simulink library "Garbage"  (R2023a) (continued)
== system Garbage/Text_Display_1x16/Character_Table/Characters(Q..ARROW_UP) ==
  Address_3_In(1b) : Inport
  Address_2_In(1b) : Inport  Port=2
  Address_1_In(1b) : Inport  Port=3
  Address_0_In(1b) : Inport  Port=4
  Byte_Multiplexer_16_to_1(Byte_0) : Byte_Multiplexers_Lib/Byte_Multiplexers_Lib/Byte_Multiplexer_16_to_1
  Byte_Multiplexer_16_to_1(Byte_1) : Byte_Multiplexers_Lib/Byte_Multiplexers_Lib/Byte_Multiplexer_16_to_1
  Byte_Multiplexer_16_to_1(Byte_2) : Byte_Multiplexers_Lib/Byte_Multiplexers_Lib/Byte_Multiplexer_16_to_1
  Byte_Multiplexer_16_to_1(Byte_3) : Byte_Multiplexers_Lib/Byte_Multiplexers_Lib/Byte_Multiplexer_16_to_1
  Byte_Multiplexer_16_to_1(Byte_4) : Byte_Multiplexers_Lib/Byte_Multiplexers_Lib/Byte_Multiplexer_16_to_1
  Byte_Multiplexer_16_to_1(Byte_5) : Byte_Multiplexers_Lib/Byte_Multiplexers_Lib/Byte_Multiplexer_16_to_1
  Byte_Multiplexer_16_to_1(Byte_6) : Byte_Multiplexers_Lib/Byte_Multiplexers_Lib/Byte_Multiplexer_16_to_1
  Byte_Multiplexer_16_to_1(Byte_7) : Byte_Multiplexers_Lib/Byte_Multiplexers_Lib/Byte_Multiplexer_16_to_1
  Char_ARROW_UP : Subsystem
  Char_ASTERISK : Subsystem
  Char_BACKSLASH : Subsystem
  Char_COLON : Subsystem
  Char_CROSS : Subsystem
  Char_EQUAL : Subsystem
  Char_GREATER_THAN : Subsystem
  Char_LESS_THAN : Subsystem
  Char_MINUS : Subsystem
  Char_PLUS : Subsystem
  Char_Q : Subsystem
  Char_SEMICOLON : Subsystem
  Char_SLASH : Subsystem
  Char_X : Subsystem
  Char_Y : Subsystem
  Char_Z : Subsystem
  Row_7(1B) : Outport
  Row_6(1B) : Outport  Port=2
  Row_5(1B) : Outport  Port=3
  Row_4(1B) : Outport  Port=4
  Row_3(1B) : Outport  Port=5
  Row_2(1B) : Outport  Port=6
  Row_1(1B) : Outport  Port=7
  Row_0(1B) : Outport  Port=8
  wires:
  Address_3_In(1b)/1 -> Byte_Multiplexer_16_to_1(Byte_0)/1, Byte_Multiplexer_16_to_1(Byte_1)/1, Byte_Multiplexer_16_to_1(Byte_2)/1, Byte_Multiplexer_16_to_1(Byte_3)/1, Byte_Multiplexer_16_to_1(Byte_4)/1, Byte_Multiplexer_16_to_1(Byte_5)/1, Byte_Multiplexer_16_to_1(Byte_6)/1, Byte_Multiplexer_16_to_1(Byte_7)/1
  Address_2_In(1b)/1 -> Byte_Multiplexer_16_to_1(Byte_0)/2, Byte_Multiplexer_16_to_1(Byte_1)/2, Byte_Multiplexer_16_to_1(Byte_2)/2, Byte_Multiplexer_16_to_1(Byte_3)/2, Byte_Multiplexer_16_to_1(Byte_4)/2, Byte_Multiplexer_16_to_1(Byte_5)/2, Byte_Multiplexer_16_to_1(Byte_6)/2, Byte_Multiplexer_16_to_1(Byte_7)/2
  Address_1_In(1b)/1 -> Byte_Multiplexer_16_to_1(Byte_0)/3, Byte_Multiplexer_16_to_1(Byte_1)/3, Byte_Multiplexer_16_to_1(Byte_2)/3, Byte_Multiplexer_16_to_1(Byte_3)/3, Byte_Multiplexer_16_to_1(Byte_4)/3, Byte_Multiplexer_16_to_1(Byte_5)/3, Byte_Multiplexer_16_to_1(Byte_6)/3, Byte_Multiplexer_16_to_1(Byte_7)/3
  Address_0_In(1b)/1 -> Byte_Multiplexer_16_to_1(Byte_0)/4, Byte_Multiplexer_16_to_1(Byte_1)/4, Byte_Multiplexer_16_to_1(Byte_2)/4, Byte_Multiplexer_16_to_1(Byte_3)/4, Byte_Multiplexer_16_to_1(Byte_4)/4, Byte_Multiplexer_16_to_1(Byte_5)/4, Byte_Multiplexer_16_to_1(Byte_6)/4, Byte_Multiplexer_16_to_1(Byte_7)/4
  Byte_Multiplexer_16_to_1(Byte_0)/1 -> Row_0(1B)/1
  Byte_Multiplexer_16_to_1(Byte_1)/1 -> Row_1(1B)/1
  Byte_Multiplexer_16_to_1(Byte_2)/1 -> Row_2(1B)/1
  Byte_Multiplexer_16_to_1(Byte_3)/1 -> Row_3(1B)/1
  Byte_Multiplexer_16_to_1(Byte_4)/1 -> Row_4(1B)/1
  Byte_Multiplexer_16_to_1(Byte_5)/1 -> Row_5(1B)/1
  Byte_Multiplexer_16_to_1(Byte_6)/1 -> Row_6(1B)/1
  Byte_Multiplexer_16_to_1(Byte_7)/1 -> Row_7(1B)/1
  Char_ARROW_UP/8(Row_0(1B)) -> Byte_Multiplexer_16_to_1(Byte_0)/5
  Char_GREATER_THAN/8(Row_0(1B)) -> Byte_Multiplexer_16_to_1(Byte_0)/6
  Char_LESS_THAN/8(Row_0(1B)) -> Byte_Multiplexer_16_to_1(Byte_0)/7
  Char_EQUAL/8(Row_0(1B)) -> Byte_Multiplexer_16_to_1(Byte_0)/8
  Char_BACKSLASH/8(Row_0(1B)) -> Byte_Multiplexer_16_to_1(Byte_0)/9
  Char_SLASH/8(Row_0(1B)) -> Byte_Multiplexer_16_to_1(Byte_0)/10
  Char_SEMICOLON/8(Row_0(1B)) -> Byte_Multiplexer_16_to_1(Byte_0)/11
  Char_COLON/8(Row_0(1B)) -> Byte_Multiplexer_16_to_1(Byte_0)/12
  Char_ASTERISK/8(Row_0(1B)) -> Byte_Multiplexer_16_to_1(Byte_0)/13
  Char_CROSS/8(Row_0(1B)) -> Byte_Multiplexer_16_to_1(Byte_0)/14
  Char_MINUS/8(Row_0(1B)) -> Byte_Multiplexer_16_to_1(Byte_0)/15
  Char_PLUS/8(Row_0(1B)) -> Byte_Multiplexer_16_to_1(Byte_0)/16
  Char_Z/8(Row_0(1B)) -> Byte_Multiplexer_16_to_1(Byte_0)/17
  Char_Y/8(Row_0(1B)) -> Byte_Multiplexer_16_to_1(Byte_0)/18
  Char_X/8(Row_0(1B)) -> Byte_Multiplexer_16_to_1(Byte_0)/19
  Char_Q/8(Row_0(1B)) -> Byte_Multiplexer_16_to_1(Byte_0)/20
  Char_ARROW_UP/7(Row_1(1B)) -> Byte_Multiplexer_16_to_1(Byte_1)/5
  Char_GREATER_THAN/7(Row_1(1B)) -> Byte_Multiplexer_16_to_1(Byte_1)/6
  Char_LESS_THAN/7(Row_1(1B)) -> Byte_Multiplexer_16_to_1(Byte_1)/7
  Char_BACKSLASH/7(Row_1(1B)) -> Byte_Multiplexer_16_to_1(Byte_1)/9
  Char_SLASH/7(Row_1(1B)) -> Byte_Multiplexer_16_to_1(Byte_1)/10
  Char_SEMICOLON/7(Row_1(1B)) -> Byte_Multiplexer_16_to_1(Byte_1)/11
  Char_COLON/7(Row_1(1B)) -> Byte_Multiplexer_16_to_1(Byte_1)/12
  Char_ASTERISK/7(Row_1(1B)) -> Byte_Multiplexer_16_to_1(Byte_1)/13
  Char_CROSS/7(Row_1(1B)) -> Byte_Multiplexer_16_to_1(Byte_1)/14
  Char_MINUS/7(Row_1(1B)) -> Byte_Multiplexer_16_to_1(Byte_1)/15
  Char_PLUS/7(Row_1(1B)) -> Byte_Multiplexer_16_to_1(Byte_1)/16
  Char_Z/7(Row_1(1B)) -> Byte_Multiplexer_16_to_1(Byte_1)/17
  Char_Y/7(Row_1(1B)) -> Byte_Multiplexer_16_to_1(Byte_1)/18
  Char_X/7(Row_1(1B)) -> Byte_Multiplexer_16_to_1(Byte_1)/19
  Char_Q/7(Row_1(1B)) -> Byte_Multiplexer_16_to_1(Byte_1)/20
  Char_ARROW_UP/6(Row_2(1B)) -> Byte_Multiplexer_16_to_1(Byte_2)/5
  Char_GREATER_THAN/6(Row_2(1B)) -> Byte_Multiplexer_16_to_1(Byte_2)/6
  Char_LESS_THAN/6(Row_2(1B)) -> Byte_Multiplexer_16_to_1(Byte_2)/7
  Char_EQUAL/6(Row_2(1B)) -> Byte_Multiplexer_16_to_1(Byte_2)/8
  Char_EQUAL/7(Row_1(1B)) -> Byte_Multiplexer_16_to_1(Byte_1)/8
  Char_BACKSLASH/6(Row_2(1B)) -> Byte_Multiplexer_16_to_1(Byte_2)/9
  Char_SLASH/6(Row_2(1B)) -> Byte_Multiplexer_16_to_1(Byte_2)/10
  Char_SEMICOLON/6(Row_2(1B)) -> Byte_Multiplexer_16_to_1(Byte_2)/11
  Char_COLON/6(Row_2(1B)) -> Byte_Multiplexer_16_to_1(Byte_2)/12
  Char_ASTERISK/6(Row_2(1B)) -> Byte_Multiplexer_16_to_1(Byte_2)/13
  Char_CROSS/6(Row_2(1B)) -> Byte_Multiplexer_16_to_1(Byte_2)/14
  Char_MINUS/6(Row_2(1B)) -> Byte_Multiplexer_16_to_1(Byte_2)/15
  Char_PLUS/6(Row_2(1B)) -> Byte_Multiplexer_16_to_1(Byte_2)/16
  Char_Z/6(Row_2(1B)) -> Byte_Multiplexer_16_to_1(Byte_2)/17
  Char_Y/6(Row_2(1B)) -> Byte_Multiplexer_16_to_1(Byte_2)/18
  Char_X/6(Row_2(1B)) -> Byte_Multiplexer_16_to_1(Byte_2)/19
  Char_Q/6(Row_2(1B)) -> Byte_Multiplexer_16_to_1(Byte_2)/20
  Char_ARROW_UP/5(Row_3(1B)) -> Byte_Multiplexer_16_to_1(Byte_3)/5
  Char_GREATER_THAN/5(Row_3(1B)) -> Byte_Multiplexer_16_to_1(Byte_3)/6
  Char_LESS_THAN/5(Row_3(1B)) -> Byte_Multiplexer_16_to_1(Byte_3)/7
  Char_EQUAL/5(Row_3(1B)) -> Byte_Multiplexer_16_to_1(Byte_3)/8
  Char_BACKSLASH/5(Row_3(1B)) -> Byte_Multiplexer_16_to_1(Byte_3)/9
  Char_SLASH/5(Row_3(1B)) -> Byte_Multiplexer_16_to_1(Byte_3)/10
  Char_SEMICOLON/5(Row_3(1B)) -> Byte_Multiplexer_16_to_1(Byte_3)/11
  Char_COLON/5(Row_3(1B)) -> Byte_Multiplexer_16_to_1(Byte_3)/12
  Char_CROSS/5(Row_3(1B)) -> Byte_Multiplexer_16_to_1(Byte_3)/14
  Char_ASTERISK/5(Row_3(1B)) -> Byte_Multiplexer_16_to_1(Byte_3)/13
  Char_MINUS/5(Row_3(1B)) -> Byte_Multiplexer_16_to_1(Byte_3)/15
  Char_PLUS/5(Row_3(1B)) -> Byte_Multiplexer_16_to_1(Byte_3)/16
  Char_Z/5(Row_3(1B)) -> Byte_Multiplexer_16_to_1(Byte_3)/17
  Char_Y/5(Row_3(1B)) -> Byte_Multiplexer_16_to_1(Byte_3)/18
  Char_X/5(Row_3(1B)) -> Byte_Multiplexer_16_to_1(Byte_3)/19
  Char_Q/5(Row_3(1B)) -> Byte_Multiplexer_16_to_1(Byte_3)/20
  Char_ARROW_UP/4(Row_4(1B)) -> Byte_Multiplexer_16_to_1(Byte_4)/5
  Char_GREATER_THAN/4(Row_4(1B)) -> Byte_Multiplexer_16_to_1(Byte_4)/6
  Char_LESS_THAN/4(Row_4(1B)) -> Byte_Multiplexer_16_to_1(Byte_4)/7
  Char_EQUAL/4(Row_4(1B)) -> Byte_Multiplexer_16_to_1(Byte_4)/8
  Char_BACKSLASH/4(Row_4(1B)) -> Byte_Multiplexer_16_to_1(Byte_4)/9
  Char_SLASH/4(Row_4(1B)) -> Byte_Multiplexer_16_to_1(Byte_4)/10
  Char_SEMICOLON/4(Row_4(1B)) -> Byte_Multiplexer_16_to_1(Byte_4)/11
  Char_COLON/4(Row_4(1B)) -> Byte_Multiplexer_16_to_1(Byte_4)/12
  Char_ASTERISK/4(Row_4(1B)) -> Byte_Multiplexer_16_to_1(Byte_4)/13
  Char_CROSS/4(Row_4(1B)) -> Byte_Multiplexer_16_to_1(Byte_4)/14
  Char_MINUS/4(Row_4(1B)) -> Byte_Multiplexer_16_to_1(Byte_4)/15
  Char_PLUS/4(Row_4(1B)) -> Byte_Multiplexer_16_to_1(Byte_4)/16
  Char_Z/4(Row_4(1B)) -> Byte_Multiplexer_16_to_1(Byte_4)/17
  Char_Y/4(Row_4(1B)) -> Byte_Multiplexer_16_to_1(Byte_4)/18
  Char_X/4(Row_4(1B)) -> Byte_Multiplexer_16_to_1(Byte_4)/19
  Char_Q/4(Row_4(1B)) -> Byte_Multiplexer_16_to_1(Byte_4)/20
  Char_ARROW_UP/3(Row_5(1B)) -> Byte_Multiplexer_16_to_1(Byte_5)/5
  Char_GREATER_THAN/3(Row_5(1B)) -> Byte_Multiplexer_16_to_1(Byte_5)/6
  Char_LESS_THAN/3(Row_5(1B)) -> Byte_Multiplexer_16_to_1(Byte_5)/7
  Char_EQUAL/3(Row_5(1B)) -> Byte_Multiplexer_16_to_1(Byte_5)/8
  Char_BACKSLASH/3(Row_5(1B)) -> Byte_Multiplexer_16_to_1(Byte_5)/9
  Char_SLASH/3(Row_5(1B)) -> Byte_Multiplexer_16_to_1(Byte_5)/10
  Char_SEMICOLON/3(Row_5(1B)) -> Byte_Multiplexer_16_to_1(Byte_5)/11
  Char_COLON/3(Row_5(1B)) -> Byte_Multiplexer_16_to_1(Byte_5)/12
  Char_ASTERISK/3(Row_5(1B)) -> Byte_Multiplexer_16_to_1(Byte_5)/13
  Char_CROSS/3(Row_5(1B)) -> Byte_Multiplexer_16_to_1(Byte_5)/14
  Char_MINUS/3(Row_5(1B)) -> Byte_Multiplexer_16_to_1(Byte_5)/15
  Char_PLUS/3(Row_5(1B)) -> Byte_Multiplexer_16_to_1(Byte_5)/16
  Char_Z/3(Row_5(1B)) -> Byte_Multiplexer_16_to_1(Byte_5)/17
  Char_Y/3(Row_5(1B)) -> Byte_Multiplexer_16_to_1(Byte_5)/18
  Char_X/3(Row_5(1B)) -> Byte_Multiplexer_16_to_1(Byte_5)/19
  Char_Q/3(Row_5(1B)) -> Byte_Multiplexer_16_to_1(Byte_5)/20
  Char_GREATER_THAN/2(Row_6(1B)) -> Byte_Multiplexer_16_to_1(Byte_6)/6
  Char_LESS_THAN/2(Row_6(1B)) -> Byte_Multiplexer_16_to_1(Byte_6)/7
  Char_EQUAL/2(Row_6(1B)) -> Byte_Multiplexer_16_to_1(Byte_6)/8
  Char_BACKSLASH/2(Row_6(1B)) -> Byte_Multiplexer_16_to_1(Byte_6)/9
  Char_SLASH/2(Row_6(1B)) -> Byte_Multiplexer_16_to_1(Byte_6)/10
  Char_SEMICOLON/2(Row_6(1B)) -> Byte_Multiplexer_16_to_1(Byte_6)/11
  Char_COLON/2(Row_6(1B)) -> Byte_Multiplexer_16_to_1(Byte_6)/12
  Char_ASTERISK/2(Row_6(1B)) -> Byte_Multiplexer_16_to_1(Byte_6)/13
  Char_CROSS/2(Row_6(1B)) -> Byte_Multiplexer_16_to_1(Byte_6)/14
  Char_MINUS/2(Row_6(1B)) -> Byte_Multiplexer_16_to_1(Byte_6)/15
  Char_PLUS/2(Row_6(1B)) -> Byte_Multiplexer_16_to_1(Byte_6)/16
  Char_Z/2(Row_6(1B)) -> Byte_Multiplexer_16_to_1(Byte_6)/17
  Char_Y/2(Row_6(1B)) -> Byte_Multiplexer_16_to_1(Byte_6)/18
  Char_X/2(Row_6(1B)) -> Byte_Multiplexer_16_to_1(Byte_6)/19
  Char_Q/2(Row_6(1B)) -> Byte_Multiplexer_16_to_1(Byte_6)/20
  Char_ARROW_UP/2(Row_6(1B)) -> Byte_Multiplexer_16_to_1(Byte_6)/5
  Char_Q/1(Row_7(1B)) -> Byte_Multiplexer_16_to_1(Byte_7)/20
  Char_X/1(Row_7(1B)) -> Byte_Multiplexer_16_to_1(Byte_7)/19
  Char_Y/1(Row_7(1B)) -> Byte_Multiplexer_16_to_1(Byte_7)/18
  Char_Z/1(Row_7(1B)) -> Byte_Multiplexer_16_to_1(Byte_7)/17
  Char_PLUS/1(Row_7(1B)) -> Byte_Multiplexer_16_to_1(Byte_7)/16
  Char_MINUS/1(Row_7(1B)) -> Byte_Multiplexer_16_to_1(Byte_7)/15
  Char_CROSS/1(Row_7(1B)) -> Byte_Multiplexer_16_to_1(Byte_7)/14
  Char_ASTERISK/1(Row_7(1B)) -> Byte_Multiplexer_16_to_1(Byte_7)/13
  Char_COLON/1(Row_7(1B)) -> Byte_Multiplexer_16_to_1(Byte_7)/12
  Char_SEMICOLON/1(Row_7(1B)) -> Byte_Multiplexer_16_to_1(Byte_7)/11
  Char_SLASH/1(Row_7(1B)) -> Byte_Multiplexer_16_to_1(Byte_7)/10
  Char_BACKSLASH/1(Row_7(1B)) -> Byte_Multiplexer_16_to_1(Byte_7)/9
  Char_EQUAL/1(Row_7(1B)) -> Byte_Multiplexer_16_to_1(Byte_7)/8
  Char_LESS_THAN/1(Row_7(1B)) -> Byte_Multiplexer_16_to_1(Byte_7)/7
  Char_GREATER_THAN/1(Row_7(1B)) -> Byte_Multiplexer_16_to_1(Byte_7)/6
  Char_ARROW_UP/1(Row_7(1B)) -> Byte_Multiplexer_16_to_1(Byte_7)/5

== system Garbage/Text_Display_1x16/Character_Table/Characters(0..F)/Char_0 ==
  Byte_01 : Constant  Value=c.char_0(:, 2)  OutDataTypeStr=uint8
  Byte_02 : Constant  Value=c.char_0(:, 3)  OutDataTypeStr=uint8
  Byte_03 : Constant  Value=c.char_0(:, 4)  OutDataTypeStr=uint8
  Byte_04 : Constant  Value=c.char_0(:, 5)  OutDataTypeStr=uint8
  Byte_05 : Constant  Value=c.char_0(:, 6)  OutDataTypeStr=uint8
  Byte_06 : Constant  Value=c.char_0(:, 7)  OutDataTypeStr=uint8
  Byte_07 : Constant  Value=c.char_0(:, 8)  OutDataTypeStr=uint8
  Byte_1 : Constant  Value=c.char_0(:, 1)  OutDataTypeStr=uint8
  Row_7(1B) : Outport
  Row_6(1B) : Outport  Port=2
  Row_5(1B) : Outport  Port=3
  Row_4(1B) : Outport  Port=4
  Row_3(1B) : Outport  Port=5
  Row_2(1B) : Outport  Port=6
  Row_1(1B) : Outport  Port=7
  Row_0(1B) : Outport  Port=8
  wires:
  Byte_02/1 -> Row_5(1B)/1
  Byte_1/1 -> Row_7(1B)/1
  Byte_06/1 -> Row_1(1B)/1
  Byte_03/1 -> Row_4(1B)/1
  Byte_07/1 -> Row_0(1B)/1
  Byte_01/1 -> Row_6(1B)/1
  Byte_04/1 -> Row_3(1B)/1
  Byte_05/1 -> Row_2(1B)/1

== system Garbage/Text_Display_1x16/Character_Table/Characters(0..F)/Char_1 ==
  Byte_01 : Constant  Value=c.char(2, 1)  OutDataTypeStr=uint8
  Byte_02 : Constant  Value=c.char(3, 1)  OutDataTypeStr=uint8
  Byte_03 : Constant  Value=c.char(4, 1)  OutDataTypeStr=uint8
  Byte_04 : Constant  Value=c.char(5, 1)  OutDataTypeStr=uint8
  Byte_05 : Constant  Value=c.char(6, 1)  OutDataTypeStr=uint8
  Byte_06 : Constant  Value=c.char(7, 1)  OutDataTypeStr=uint8
  Byte_07 : Constant  Value=c.char(8, 1)  OutDataTypeStr=uint8
  Byte_1 : Constant  Value=c.char(1, 1)  OutDataTypeStr=uint8
  Row_7(1B) : Outport
  Row_6(1B) : Outport  Port=2
  Row_5(1B) : Outport  Port=3
  Row_4(1B) : Outport  Port=4
  Row_3(1B) : Outport  Port=5
  Row_2(1B) : Outport  Port=6
  Row_1(1B) : Outport  Port=7
  Row_0(1B) : Outport  Port=8
  wires:
  Byte_02/1 -> Row_5(1B)/1
  Byte_1/1 -> Row_7(1B)/1
  Byte_06/1 -> Row_1(1B)/1
  Byte_03/1 -> Row_4(1B)/1
  Byte_07/1 -> Row_0(1B)/1
  Byte_01/1 -> Row_6(1B)/1
  Byte_04/1 -> Row_3(1B)/1
  Byte_05/1 -> Row_2(1B)/1

== system Garbage/Text_Display_1x16/Character_Table/Characters(0..F)/Char_2 ==
  Byte_01 : Constant  Value=c.char(2, 2)  OutDataTypeStr=uint8
  Byte_02 : Constant  Value=c.char(3, 2)  OutDataTypeStr=uint8
  Byte_03 : Constant  Value=c.char(4, 2)  OutDataTypeStr=uint8
  Byte_04 : Constant  Value=c.char(5, 2)  OutDataTypeStr=uint8
  Byte_05 : Constant  Value=c.char(6, 2)  OutDataTypeStr=uint8
  Byte_06 : Constant  Value=c.char(7, 2)  OutDataTypeStr=uint8
  Byte_07 : Constant  Value=c.char(8, 2)  OutDataTypeStr=uint8
  Byte_1 : Constant  Value=c.char(1, 2)  OutDataTypeStr=uint8
  Row_7(1B) : Outport
  Row_6(1B) : Outport  Port=2
  Row_5(1B) : Outport  Port=3
  Row_4(1B) : Outport  Port=4
  Row_3(1B) : Outport  Port=5
  Row_2(1B) : Outport  Port=6
  Row_1(1B) : Outport  Port=7
  Row_0(1B) : Outport  Port=8
  wires:
  Byte_02/1 -> Row_5(1B)/1
  Byte_1/1 -> Row_7(1B)/1
  Byte_06/1 -> Row_1(1B)/1
  Byte_03/1 -> Row_4(1B)/1
  Byte_07/1 -> Row_0(1B)/1
  Byte_01/1 -> Row_6(1B)/1
  Byte_04/1 -> Row_3(1B)/1
  Byte_05/1 -> Row_2(1B)/1

== system Garbage/Text_Display_1x16/Character_Table/Characters(0..F)/Char_3 ==
  Byte_01 : Constant  Value=c.char(2, 3)  OutDataTypeStr=uint8
  Byte_02 : Constant  Value=c.char(3, 3)  OutDataTypeStr=uint8
  Byte_03 : Constant  Value=c.char(4, 3)  OutDataTypeStr=uint8
  Byte_04 : Constant  Value=c.char(5, 3)  OutDataTypeStr=uint8
  Byte_05 : Constant  Value=c.char(6, 3)  OutDataTypeStr=uint8
  Byte_06 : Constant  Value=c.char(7, 3)  OutDataTypeStr=uint8
  Byte_07 : Constant  Value=c.char(8, 3)  OutDataTypeStr=uint8
  Byte_1 : Constant  Value=c.char(1, 3)  OutDataTypeStr=uint8
  Row_7(1B) : Outport
  Row_6(1B) : Outport  Port=2
  Row_5(1B) : Outport  Port=3
  Row_4(1B) : Outport  Port=4
  Row_3(1B) : Outport  Port=5
  Row_2(1B) : Outport  Port=6
  Row_1(1B) : Outport  Port=7
  Row_0(1B) : Outport  Port=8
  wires:
  Byte_02/1 -> Row_5(1B)/1
  Byte_1/1 -> Row_7(1B)/1
  Byte_06/1 -> Row_1(1B)/1
  Byte_03/1 -> Row_4(1B)/1
  Byte_07/1 -> Row_0(1B)/1
  Byte_01/1 -> Row_6(1B)/1
  Byte_04/1 -> Row_3(1B)/1
  Byte_05/1 -> Row_2(1B)/1

== system Garbage/Text_Display_1x16/Character_Table/Characters(0..F)/Char_4 ==
  Byte_01 : Constant  Value=c.char(2, 4)  OutDataTypeStr=uint8
  Byte_02 : Constant  Value=c.char(3, 4)  OutDataTypeStr=uint8
  Byte_03 : Constant  Value=c.char(4, 4)  OutDataTypeStr=uint8
  Byte_04 : Constant  Value=c.char(5, 4)  OutDataTypeStr=uint8
  Byte_05 : Constant  Value=c.char(6, 4)  OutDataTypeStr=uint8
  Byte_06 : Constant  Value=c.char(7, 4)  OutDataTypeStr=uint8
  Byte_07 : Constant  Value=c.char(8, 4)  OutDataTypeStr=uint8
  Byte_1 : Constant  Value=c.char(1, 4)  OutDataTypeStr=uint8
  Row_7(1B) : Outport
  Row_6(1B) : Outport  Port=2
  Row_5(1B) : Outport  Port=3
  Row_4(1B) : Outport  Port=4
  Row_3(1B) : Outport  Port=5
  Row_2(1B) : Outport  Port=6
  Row_1(1B) : Outport  Port=7
  Row_0(1B) : Outport  Port=8
  wires:
  Byte_02/1 -> Row_5(1B)/1
  Byte_1/1 -> Row_7(1B)/1
  Byte_06/1 -> Row_1(1B)/1
  Byte_03/1 -> Row_4(1B)/1
  Byte_07/1 -> Row_0(1B)/1
  Byte_01/1 -> Row_6(1B)/1
  Byte_04/1 -> Row_3(1B)/1
  Byte_05/1 -> Row_2(1B)/1

== system Garbage/Text_Display_1x16/Character_Table/Characters(0..F)/Char_5 ==
  Byte_01 : Constant  Value=c.char(2, 5)  OutDataTypeStr=uint8
  Byte_02 : Constant  Value=c.char(3, 5)  OutDataTypeStr=uint8
  Byte_03 : Constant  Value=c.char(4, 5)  OutDataTypeStr=uint8
  Byte_04 : Constant  Value=c.char(5, 5)  OutDataTypeStr=uint8
  Byte_05 : Constant  Value=c.char(6, 5)  OutDataTypeStr=uint8
  Byte_06 : Constant  Value=c.char(7, 5)  OutDataTypeStr=uint8
  Byte_07 : Constant  Value=c.char(8, 5)  OutDataTypeStr=uint8
  Byte_1 : Constant  Value=c.char(1, 5)  OutDataTypeStr=uint8
  Row_7(1B) : Outport
  Row_6(1B) : Outport  Port=2
  Row_5(1B) : Outport  Port=3
  Row_4(1B) : Outport  Port=4
  Row_3(1B) : Outport  Port=5
  Row_2(1B) : Outport  Port=6
  Row_1(1B) : Outport  Port=7
  Row_0(1B) : Outport  Port=8
  wires:
  Byte_02/1 -> Row_5(1B)/1
  Byte_1/1 -> Row_7(1B)/1
  Byte_06/1 -> Row_1(1B)/1
  Byte_03/1 -> Row_4(1B)/1
  Byte_07/1 -> Row_0(1B)/1
  Byte_01/1 -> Row_6(1B)/1
  Byte_04/1 -> Row_3(1B)/1
  Byte_05/1 -> Row_2(1B)/1

== system Garbage/Text_Display_1x16/Character_Table/Characters(0..F)/Char_6 ==
  Byte_01 : Constant  Value=c.char(2, 6)  OutDataTypeStr=uint8
  Byte_02 : Constant  Value=c.char(3, 6)  OutDataTypeStr=uint8
  Byte_03 : Constant  Value=c.char(4, 6)  OutDataTypeStr=uint8
  Byte_04 : Constant  Value=c.char(5, 6)  OutDataTypeStr=uint8
  Byte_05 : Constant  Value=c.char(6, 6)  OutDataTypeStr=uint8
  Byte_06 : Constant  Value=c.char(7, 6)  OutDataTypeStr=uint8
  Byte_07 : Constant  Value=c.char(8, 6)  OutDataTypeStr=uint8
  Byte_1 : Constant  Value=c.char(1, 6)  OutDataTypeStr=uint8
  Row_7(1B) : Outport
  Row_6(1B) : Outport  Port=2
  Row_5(1B) : Outport  Port=3
  Row_4(1B) : Outport  Port=4
  Row_3(1B) : Outport  Port=5
  Row_2(1B) : Outport  Port=6
  Row_1(1B) : Outport  Port=7
  Row_0(1B) : Outport  Port=8
  wires:
  Byte_02/1 -> Row_5(1B)/1
  Byte_1/1 -> Row_7(1B)/1
  Byte_06/1 -> Row_1(1B)/1
  Byte_03/1 -> Row_4(1B)/1
  Byte_07/1 -> Row_0(1B)/1
  Byte_01/1 -> Row_6(1B)/1
  Byte_04/1 -> Row_3(1B)/1
  Byte_05/1 -> Row_2(1B)/1

== system Garbage/Text_Display_1x16/Character_Table/Characters(0..F)/Char_7 ==
  Byte_01 : Constant  Value=c.char(2, 7)  OutDataTypeStr=uint8
  Byte_02 : Constant  Value=c.char(3, 7)  OutDataTypeStr=uint8
  Byte_03 : Constant  Value=c.char(4, 7)  OutDataTypeStr=uint8
  Byte_04 : Constant  Value=c.char(5, 7)  OutDataTypeStr=uint8
  Byte_05 : Constant  Value=c.char(6, 7)  OutDataTypeStr=uint8
  Byte_06 : Constant  Value=c.char(7, 7)  OutDataTypeStr=uint8
  Byte_07 : Constant  Value=c.char(8, 7)  OutDataTypeStr=uint8
  Byte_1 : Constant  Value=c.char(1, 7)  OutDataTypeStr=uint8
  Row_7(1B) : Outport
  Row_6(1B) : Outport  Port=2
  Row_5(1B) : Outport  Port=3
  Row_4(1B) : Outport  Port=4
  Row_3(1B) : Outport  Port=5
  Row_2(1B) : Outport  Port=6
  Row_1(1B) : Outport  Port=7
  Row_0(1B) : Outport  Port=8
  wires:
  Byte_02/1 -> Row_5(1B)/1
  Byte_1/1 -> Row_7(1B)/1
  Byte_06/1 -> Row_1(1B)/1
  Byte_03/1 -> Row_4(1B)/1
  Byte_07/1 -> Row_0(1B)/1
  Byte_01/1 -> Row_6(1B)/1
  Byte_04/1 -> Row_3(1B)/1
  Byte_05/1 -> Row_2(1B)/1

== system Garbage/Text_Display_1x16/Character_Table/Characters(0..F)/Char_8 ==
  Byte_01 : Constant  Value=c.char(2, 8)  OutDataTypeStr=uint8
  Byte_02 : Constant  Value=c.char(3, 8)  OutDataTypeStr=uint8
  Byte_03 : Constant  Value=c.char(4, 8)  OutDataTypeStr=uint8
  Byte_04 : Constant  Value=c.char(5, 8)  OutDataTypeStr=uint8
  Byte_05 : Constant  Value=c.char(6, 8)  OutDataTypeStr=uint8
  Byte_06 : Constant  Value=c.char(7, 8)  OutDataTypeStr=uint8
  Byte_07 : Constant  Value=c.char(8, 8)  OutDataTypeStr=uint8
  Byte_1 : Constant  Value=c.char(1, 8)  OutDataTypeStr=uint8
  Row_7(1B) : Outport
  Row_6(1B) : Outport  Port=2
  Row_5(1B) : Outport  Port=3
  Row_4(1B) : Outport  Port=4
  Row_3(1B) : Outport  Port=5
  Row_2(1B) : Outport  Port=6
  Row_1(1B) : Outport  Port=7
  Row_0(1B) : Outport  Port=8
  wires:
  Byte_02/1 -> Row_5(1B)/1
  Byte_1/1 -> Row_7(1B)/1
  Byte_06/1 -> Row_1(1B)/1
  Byte_03/1 -> Row_4(1B)/1
  Byte_07/1 -> Row_0(1B)/1
  Byte_01/1 -> Row_6(1B)/1
  Byte_04/1 -> Row_3(1B)/1
  Byte_05/1 -> Row_2(1B)/1

== system Garbage/Text_Display_1x16/Character_Table/Characters(0..F)/Char_9 ==
  Byte_01 : Constant  Value=c.char(2, 9)  OutDataTypeStr=uint8
  Byte_02 : Constant  Value=c.char(3, 9)  OutDataTypeStr=uint8
  Byte_03 : Constant  Value=c.char(4, 9)  OutDataTypeStr=uint8
  Byte_04 : Constant  Value=c.char(5, 9)  OutDataTypeStr=uint8
  Byte_05 : Constant  Value=c.char(6, 9)  OutDataTypeStr=uint8
  Byte_06 : Constant  Value=c.char(7, 9)  OutDataTypeStr=uint8
  Byte_07 : Constant  Value=c.char(8, 9)  OutDataTypeStr=uint8
  Byte_1 : Constant  Value=c.char(1, 9)  OutDataTypeStr=uint8
  Row_7(1B) : Outport
  Row_6(1B) : Outport  Port=2
  Row_5(1B) : Outport  Port=3
  Row_4(1B) : Outport  Port=4
  Row_3(1B) : Outport  Port=5
  Row_2(1B) : Outport  Port=6
  Row_1(1B) : Outport  Port=7
  Row_0(1B) : Outport  Port=8
  wires:
  Byte_02/1 -> Row_5(1B)/1
  Byte_1/1 -> Row_7(1B)/1
  Byte_06/1 -> Row_1(1B)/1
  Byte_03/1 -> Row_4(1B)/1
  Byte_07/1 -> Row_0(1B)/1
  Byte_01/1 -> Row_6(1B)/1
  Byte_04/1 -> Row_3(1B)/1
  Byte_05/1 -> Row_2(1B)/1

== system Garbage/Text_Display_1x16/Character_Table/Characters(0..F)/Char_A ==
  Byte_01 : Constant  Value=c.char(2, c.A)  OutDataTypeStr=uint8
  Byte_02 : Constant  Value=c.char(3, c.A)  OutDataTypeStr=uint8
  Byte_03 : Constant  Value=c.char(4, c.A)  OutDataTypeStr=uint8
  Byte_04 : Constant  Value=c.char(5, c.A)  OutDataTypeStr=uint8
  Byte_05 : Constant  Value=c.char(6, c.A)  OutDataTypeStr=uint8
  Byte_06 : Constant  Value=c.char(7, c.A)  OutDataTypeStr=uint8
  Byte_07 : Constant  Value=c.char(8, c.A)  OutDataTypeStr=uint8
  Byte_1 : Constant  Value=c.char(1, c.A)  OutDataTypeStr=uint8
  Row_7(1B) : Outport
  Row_6(1B) : Outport  Port=2
  Row_5(1B) : Outport  Port=3
  Row_4(1B) : Outport  Port=4
  Row_3(1B) : Outport  Port=5
  Row_2(1B) : Outport  Port=6
  Row_1(1B) : Outport  Port=7
  Row_0(1B) : Outport  Port=8
  wires:
  Byte_02/1 -> Row_5(1B)/1
  Byte_1/1 -> Row_7(1B)/1
  Byte_06/1 -> Row_1(1B)/1
  Byte_03/1 -> Row_4(1B)/1
  Byte_07/1 -> Row_0(1B)/1
  Byte_01/1 -> Row_6(1B)/1
  Byte_04/1 -> Row_3(1B)/1
  Byte_05/1 -> Row_2(1B)/1

== system Garbage/Text_Display_1x16/Character_Table/Characters(0..F)/Char_B ==
  Byte_01 : Constant  Value=c.char(2, c.B)  OutDataTypeStr=uint8
  Byte_02 : Constant  Value=c.char(3, c.B)  OutDataTypeStr=uint8
  Byte_03 : Constant  Value=c.char(4, c.B)  OutDataTypeStr=uint8
  Byte_04 : Constant  Value=c.char(5, c.B)  OutDataTypeStr=uint8
  Byte_05 : Constant  Value=c.char(6, c.B)  OutDataTypeStr=uint8
  Byte_06 : Constant  Value=c.char(7, c.B)  OutDataTypeStr=uint8
  Byte_07 : Constant  Value=c.char(8, c.B)  OutDataTypeStr=uint8
  Byte_1 : Constant  Value=c.char(1, c.B)  OutDataTypeStr=uint8
  Row_7(1B) : Outport
  Row_6(1B) : Outport  Port=2
  Row_5(1B) : Outport  Port=3
  Row_4(1B) : Outport  Port=4
  Row_3(1B) : Outport  Port=5
  Row_2(1B) : Outport  Port=6
  Row_1(1B) : Outport  Port=7
  Row_0(1B) : Outport  Port=8
  wires:
  Byte_02/1 -> Row_5(1B)/1
  Byte_1/1 -> Row_7(1B)/1
  Byte_06/1 -> Row_1(1B)/1
  Byte_03/1 -> Row_4(1B)/1
  Byte_07/1 -> Row_0(1B)/1
  Byte_01/1 -> Row_6(1B)/1
  Byte_04/1 -> Row_3(1B)/1
  Byte_05/1 -> Row_2(1B)/1

== system Garbage/Text_Display_1x16/Character_Table/Characters(0..F)/Char_C ==
  Byte_01 : Constant  Value=c.char(2, c.C)  OutDataTypeStr=uint8
  Byte_02 : Constant  Value=c.char(3, c.C)  OutDataTypeStr=uint8
  Byte_03 : Constant  Value=c.char(4, c.C)  OutDataTypeStr=uint8
  Byte_04 : Constant  Value=c.char(5, c.C)  OutDataTypeStr=uint8
  Byte_05 : Constant  Value=c.char(6, c.C)  OutDataTypeStr=uint8
  Byte_06 : Constant  Value=c.char(7, c.C)  OutDataTypeStr=uint8
  Byte_07 : Constant  Value=c.char(8, c.C)  OutDataTypeStr=uint8
  Byte_1 : Constant  Value=c.char(1, c.C)  OutDataTypeStr=uint8
  Row_7(1B) : Outport
  Row_6(1B) : Outport  Port=2
  Row_5(1B) : Outport  Port=3
  Row_4(1B) : Outport  Port=4
  Row_3(1B) : Outport  Port=5
  Row_2(1B) : Outport  Port=6
  Row_1(1B) : Outport  Port=7
  Row_0(1B) : Outport  Port=8
  wires:
  Byte_02/1 -> Row_5(1B)/1
  Byte_1/1 -> Row_7(1B)/1
  Byte_06/1 -> Row_1(1B)/1
  Byte_03/1 -> Row_4(1B)/1
  Byte_07/1 -> Row_0(1B)/1
  Byte_01/1 -> Row_6(1B)/1
  Byte_04/1 -> Row_3(1B)/1
  Byte_05/1 -> Row_2(1B)/1
